simulink model "TosAeb"  (R2021a)
settings: Solver=FixedStepDiscrete  StartTime=0.0  StopTime=inf  FixedStep=0.01  RelTol=1e-3
undefined symbols (not defined in the file or in its folder): ACCModSts, AccSts1, AEB_ObjectMovingOrientation, AEB_ObjectMovingState, CN_BTCActive, CN_CurrentGearR, CN_CurrentGearR_DHT, CN_PTCActive, CN_VDCActive, CN_VSC_EspOff, CN_VSC_FCW_AEB_Error, CN_VSC_FCW_AEB_NoDispay, CN_VSC_FCW_AEB_PerfmDgdtn, CN_VSC_FCW_AEB_TempUnAvlbl, CN_VSC_VehStandstill, CNaccelAvoidMax, CNaccelAvoidMin, CNbrakePedalPress, CNcomfortStrLeftAccelMax, CNcomfortStrLeftAccelMin, CNcomfortStrRightAccelMax, CNcomfortStrRightAccelMin, CNDrvDoorOpen, CNDrvModeEco, CNDrvModeNorm, CNDrvModeOther, CNDrvModeSport, CNDrvSBRreminder, CNNull, CNobjInvalidID, CNPassDoorOpen, CNPassSBRreminder, CNPEBSAccelNegInf, CNPEBSAccelNull, CNPEBSTimeInf, CNPEBSTimeNull, CNSTOPMAX, CNSTOPNULL, CNsubjWidth, CNSwtOFF, CNSwtON, CNTTCMAX, CNTTCNULL, CNVehConfigAEBCycNone, CNVehConfigAEBNone, CNVehConfigAEBPedNone, CNVehConfigFCWNone, CNVehConfigJANone, CollisionSeverity, FALSE, FCWSnvty, JACycObjCalcInfoDefaultParams, JAPedObjCalcInfoDefaultParams, JAVehObjCalcInfoDefaultParams, latCycObjCalcInfoDefaultParams, latPedObjCalcInfoDefaultParams, longObjCalcInfoDefaultParams, ModSts, movingStatus, ObjectType ... (+1112)
libraries: simulink x3031, AEBSFunctionLib x50, Ta_Library x33, AEBSStateMachineLib x23, Mylibrary x12, simulink_extras x7, ObjSelect_lib x3, dspstat3 x2
(part 37 of 102)

== system TosAeb/AEB_Objsel/TA_Processor/PreProcessor/VehiclePreProcessor/PathEstimn_Calculate/For Iterator PahEstimn/ComputeVehicleStateAtT1/c ==
  P1Longitudinal : Inport
  P2Longitudinal : Inport  Port=2
  P3Longitudinal : Inport  Port=3
  P1Lateral : Inport  Port=4
  P2Lateral : Inport  Port=5
  P3Lateral : Inport  Port=6
  Add : Sum  Inputs=-+
  Add1 : Sum  Inputs=+-+
  Add2 : Sum  Inputs=-+
  Add3 : Sum  Inputs=+-+
  Gain{,1,5..6} (4 alike) : Gain  Gain=3
  Gain2 : Gain  Gain=6
  Gain3 : Gain  Gain=12
  Gain4 : Gain  Gain=6
  Gain7 : Gain  Gain=6
  Gain8 : Gain  Gain=12
  Gain9 : Gain
  Terminator : Terminator
  Terminator1 : Terminator
  PosnLgt : Outport
  PosnLat : Outport  Port=2
  VLgt : Outport  Port=3
  VLat : Outport  Port=4
  wires:
  P3Longitudinal/1 -> [P3Longitudinal], PosnLgt/1  "PosnLgt"
  P2Longitudinal/1 -> [P2Longitudinal], Gain/1
  Gain/1 -> Add/1
  Gain1/1 -> Add/2
  [P3Longitudinal] -> Gain1/1
  Add/1 -> VLgt/1  "VLgt"
  P1Longitudinal/1 -> Gain2/1
  Gain2/1 -> Add1/1
  [P2Longitudinal] -> Gain3/1
  Gain3/1 -> Add1/2
  Gain4/1 -> Add1/3
  [P3Longitudinal] -> Gain4/1
  Add1/1 -> Terminator/1  "ALgt"
  P3Lateral/1 -> [P3Lateral], PosnLat/1
  P2Lateral/1 -> [P2Lateral], Gain5/1
  Gain5/1 -> Add2/1
  Add2/1 -> VLat/1  "VLat"
  [P3Lateral] -> Gain6/1
  Gain6/1 -> Add2/2
  P1Lateral/1 -> Gain7/1
  Gain7/1 -> Add3/1
  [P2Lateral] -> Gain8/1
  Gain8/1 -> Add3/2
  Gain9/1 -> Add3/3
  [P3Lateral] -> Gain9/1
  Add3/1 -> Terminator1/1  "ALgt"

== system TosAeb/AEB_Objsel/TA_Processor/PreProcessor/VehiclePreProcessor/PathEstimn_Calculate/For Iterator PahEstimn/InitialControlPointsCalculation/InitialControlPointsCalculation ==
  PositionLongitudinal : Inport
  VelocityLongitudinal : Inport  Port=2
  AccelerationLongitudinal : Inport  Port=3
  PositionLateral : Inport  Port=4
  VelocityLateral : Inport  Port=5
  AccelerationLateral : Inport  Port=6
  StoppingDistances : Inport  Port=7
  Add{,1..2,4} (4 alike) : Sum
  Add3 : Sum  Inputs=+-+
  Add5 : Sum  Inputs=+-+
  Bus Creator : BusCreator  Inputs=6
  Bus Selector : BusSelector  OutputSignals=StopsWithinSegment,LongitudinalStoppingDistance,LateralStoppingDistance
  Gain : Gain  Gain=single(1/3)
  Gain1 : Gain  Gain=2
  Gain2 : Gain  Gain=single(1/3)
  Gain3 : Gain  Gain=single(1/6)
  Gain4 : Gain  Gain=single(1/6)
  Gain5 : Gain  Gain=2
  Signal Conversion : SignalConversion  OverrideOpt=off
  Signal Conversion1 : SignalConversion  OverrideOpt=off
  Switch{,1..3} (4 alike) : Switch  Criteria=u2 ~= 0
  InitialControlPoints : Outport
  wires:
  PositionLongitudinal/1 -> [PosnLgt], Add/1, Signal Conversion/1
  VelocityLongitudinal/1 -> Gain/1
  Gain/1 -> Add/2
  Add/1 -> Gain1/1, Switch/3
  StoppingDistances/1 -> Bus Selector/1
  Bus Selector/1 -> [StopsWithinSegment]  "<StopsWithinSegment>"
  Bus Selector/2 -> Add1/1  "<LongitudinalStoppingDistance>"
  [PosnLgt] -> Add1/2
  Add1/1 -> [StopPosnLgt]
  Bus Selector/3 -> Add2/1  "<LateralStoppingDistance>"
  PositionLateral/1 -> Add4/1, Signal Conversion1/1, [PosnLat]
  [PosnLat] -> Add2/2
  Add2/1 -> [StopPosnLat]
  [StopPosnLgt] -> Switch/1
  [StopsWithinSegment] -> Switch/2
  Gain1/1 -> Add3/1
  [PosnLgt] -> Add3/2
  Add3/1 -> Switch1/3
  [StopPosnLgt] -> Switch1/1
  [StopsWithinSegment] -> Switch1/2
  AccelerationLongitudinal/1 -> Gain3/1
  Gain3/1 -> Add3/3
  Gain2/1 -> Add4/2
  VelocityLateral/1 -> Gain2/1
  AccelerationLateral/1 -> Gain4/1
  Gain4/1 -> Add5/3
  [PosnLat] -> Add5/2
  Add4/1 -> Switch3/3, Gain5/1
  Add5/1 -> Switch2/3
  [StopPosnLat] -> Switch2/1
  [StopsWithinSegment] -> Switch2/2
  Gain5/1 -> Add5/1
  [StopPosnLat] -> Switch3/1
  [StopsWithinSegment] -> Switch3/2
  Signal Conversion/1 -> Bus Creator/1  "P0Longitudinal"
  Switch2/1 -> Bus Creator/6  "P2Lateral"
  Switch/1 -> Bus Creator/2  "P1Longitudinal"
  Switch1/1 -> Bus Creator/3  "P2Longitudinal"
  Signal Conversion1/1 -> Bus Creator/4  "P0Lateral"
  Switch3/1 -> Bus Creator/5  "P1Lateral"
  Bus Creator/1 -> InitialControlPoints/1  "InitialControlPoints"

== system TosAeb/FcwAebJa_Step_sys/ALG_AEBSystem/ALG_AEBController/AEB_FuncCondCalcApp/CycFunctionCondCalc/cycAEBfullSuppAborCond/cycAEBfullAborCondNoStandstillCalc/cycAEBfullAborCondFinalJudge ==
  cycAEBfullObjRelAborCondFulfilled : Inport
  cycAEBfullVehRelAborCondFulfilled : Inport  Port=2
  cycAEBfullSituationRelAborCondFulfilled : Inport  Port=3
  Compare To Constant1 : simulink/Logic and Bit Operations/Compare To Constant  relop===  const=TRUE  OutDataTypeStr=boolean
  Compare To Constant2 : simulink/Logic and Bit Operations/Compare To Constant  relop===  const=TRUE  OutDataTypeStr=boolean
  Compare To Constant3 : simulink/Logic and Bit Operations/Compare To Constant  relop===  const=TRUE  OutDataTypeStr=boolean
  Logical Operator : Logic  Operator=OR  Inputs=3  AllPortsSameDT=off  OutDataTypeStr=boolean
  cycAEBfullAborCondNoStandstillFulfilled : Outport
  wires:
  Compare To Constant3/1 -> Logical Operator/1
  cycAEBfullVehRelAborCondFulfilled/1 -> Compare To Constant1/1
  cycAEBfullSituationRelAborCondFulfilled/1 -> Compare To Constant2/1
  Logical Operator/1 -> cycAEBfullAborCondNoStandstillFulfilled/1  "cycAEBfullAborCondNoStandstillFulfilled"
  cycAEBfullObjRelAborCondFulfilled/1 -> Compare To Constant3/1
  Compare To Constant1/1 -> Logical Operator/2
  Compare To Constant2/1 -> Logical Operator/3

== system TosAeb/FcwAebJa_Step_sys/ALG_AEBSystem/ALG_AEBController/AEB_FuncCondCalcApp/CycFunctionCondCalc/cycAEBfullSuppAborCond/cycAEBfullAborCondNoStandstillCalc/cycAEBfullObjRelActiveCondCalc ==
  ObjectID : Inport
  objIsFusionTarget : Inport  Port=2
  latCollsionRiskProb : Inport  Port=3
  Chart : Stateflow chart  TreatAsAtomicUnit=on
  Compare To Constant : simulink/Logic and Bit Operations/Compare To Constant  relop===  const=TRUE  OutDataTypeStr=boolean
  Constant : Constant  Value=P_AEB_Func_cycAEBfulColliisonRiskProbAborThresObjID
  Unit Delay : UnitDelay  SampleTime=-1  HasFrameUpgradeWarning=on
  cycAEBfullObjRelAborCondFulfilled : Outport
  wires:
  Constant/1 -> Chart/5(P_cycAEBfulColliisonRiskProbAborThresObjID)
  latCollsionRiskProb/1 -> Chart/4(objLatCollisionProb)
  Unit Delay/1 -> Chart/2(targetObjIDObjCalcK1)
  Chart/1(cycAEBfullObjRelAborCondFulfilled) -> cycAEBfullObjRelAborCondFulfilled/1
  ObjectID/1 -> Unit Delay/1, Chart/1(targetObjID)
  objIsFusionTarget/1 -> Compare To Constant/1
  Compare To Constant/1 -> Chart/3(objIsFusionTarget)

== system TosAeb/FcwAebJa_Step_sys/ALG_AEBSystem/ALG_AEBController/AEB_FuncCondCalcApp/CycFunctionCondCalc/cycAEBfullSuppAborCond/cycAEBfullAborCondNoStandstillCalc/cycAEBfullSituationRelAborCondCalc ==
  cycVehVelReduced : Inport
  cycCollisionSeverity : Inport  Port=2
  objLatCollsionRiskProb : Inport  Port=3
  subjVel : Inport  Port=4
  dy : Inport  Port=5
  dx : Inport  Port=6
  ObjectMovingState : Inport  Port=7
  ObjLatMovContiInvalidCalc : Subsystem
  Subsystem : Subsystem
  cycAEBfullSituationRelAborCondFulfilled : Outport
  wires:
  ObjectMovingState/1 -> ObjLatMovContiInvalidCalc/4(ObjectMovingState)
  dx/1 -> ObjLatMovContiInvalidCalc/3(dx)
  dy/1 -> ObjLatMovContiInvalidCalc/2(dy)
  subjVel/1 -> ObjLatMovContiInvalidCalc/1(subjVel)
  Subsystem/1(cycAEBfullSituationRelAborCondFulfilled) -> cycAEBfullSituationRelAborCondFulfilled/1
  ObjLatMovContiInvalidCalc/1(ObjLatMovContiInvalid) -> Subsystem/4(ObjLatMovContiInvalid)
  objLatCollsionRiskProb/1 -> Subsystem/3(objLatCollsionRiskProb)
  cycVehVelReduced/1 -> Subsystem/2(cycVehVelReduced)
  cycCollisionSeverity/1 -> Subsystem/1(cycCollisionSeverity)

== system TosAeb/FcwAebJa_Step_sys/ALG_AEBSystem/ALG_AEBController/AEB_FuncCondCalcApp/CycFunctionCondCalc/cycAEBfullSuppAborCond/cycAEBfullAborCondNoStandstillCalc/cycAEBfullVehRelAborCondCalc ==
  note: 取消自车速度判断条件
  SteerWheelAngle : Inport
  SteerWheelSpd : Inport  Port=2
  vehStateCalcAEBfullAborFlg : Inport  Port=3
  cycAEBfullWorkTime : Inport  Port=4
  vehStateCalcStandstill : Inport  Port=5
  Abs : Abs
  Abs1 : Abs
  Compare To Constant : simulink/Logic and Bit Operations/Compare To Constant  relop=>  const=P_AEB_Func_cycAEBfullStrWheelAngleAborThres  OutDataTypeStr=boolean
  Compare To Constant1 : simulink/Logic and Bit Operations/Compare To Constant  relop=>  const=P_AEB_Func_cycAEBfullStrWheelAngleSpdAborThres  OutDataTypeStr=boolean
  Compare To Constant2 : simulink/Logic and Bit Operations/Compare To Constant  relop=>  const=P_AEB_Func_cycAEBfullActivationTimeAborThres  OutDataTypeStr=boolean
  Compare To Constant3 : simulink/Logic and Bit Operations/Compare To Constant  relop===  const=TRUE  OutDataTypeStr=boolean
  Compare To Constant4 : simulink/Logic and Bit Operations/Compare To Constant  relop===  const=FALSE  OutDataTypeStr=boolean
  Logical Operator : Logic  Operator=OR  AllPortsSameDT=off  OutDataTypeStr=boolean
  Logical Operator2 : Logic  Operator=OR  Inputs=3  AllPortsSameDT=off  OutDataTypeStr=boolean
  Logical Operator4 : Logic  AllPortsSameDT=off  OutDataTypeStr=boolean
  cycAEBfullVehRelAborCondFulfilled : Outport
  wires:
  Compare To Constant2/1 -> Logical Operator4/2
  Compare To Constant4/1 -> Logical Operator4/1
  vehStateCalcStandstill/1 -> Compare To Constant4/1
  Logical Operator2/1 -> cycAEBfullVehRelAborCondFulfilled/1  "cycAEBfullVehRelAborCondFulfilled"
  Logical Operator/1 -> Logical Operator2/1  "strReleventAborCondFulfilled"
  Compare To Constant/1 -> Logical Operator/1
  vehStateCalcAEBfullAborFlg/1 -> Compare To Constant3/1
  cycAEBfullWorkTime/1 -> Compare To Constant2/1
  SteerWheelAngle/1 -> Abs/1
  Compare To Constant1/1 -> Logical Operator/2
  Abs1/1 -> Compare To Constant1/1
  SteerWheelSpd/1 -> Abs1/1
  Abs/1 -> Compare To Constant/1
  Logical Operator4/1 -> Logical Operator2/3  "cycAEBfullWorkTimeRelCondFulfilled"
  Compare To Constant3/1 -> Logical Operator2/2  "vehStateCalcAEBfullAborFlg"

== system TosAeb/FcwAebJa_Step_sys/ALG_AEBSystem/ALG_AEBController/AEB_FuncCondCalcApp/CycFunctionCondCalc/cycAEBfullSuppAborCond/cycAEBfullAborCondStandstillCalc/cycAEBfullVehRelAborCondCalc ==
  note: 取消速度限制
  subjVel : Inport
  SteerWheelAngle : Inport  Port=2
  SteerWheelSpd : Inport  Port=3
  vehStateCalcAEBfullAborFlg : Inport  Port=4
  cycAEBfullWorkTime : Inport  Port=5
  vehStateCalcStandstill : Inport  Port=6
  Abs : Abs
  Abs1 : Abs
  Compare To Constant : simulink/Logic and Bit Operations/Compare To Constant  relop=>  const=1.57  OutDataTypeStr=boolean
  Compare To Constant1 : simulink/Logic and Bit Operations/Compare To Constant  relop=>  const=1.75  OutDataTypeStr=boolean
  Compare To Constant2 : simulink/Logic and Bit Operations/Compare To Constant  relop=>  const=3.5  OutDataTypeStr=boolean
  Compare To Constant3 : simulink/Logic and Bit Operations/Compare To Constant  relop===  const=TRUE  OutDataTypeStr=boolean
  Compare To Constant4 : simulink/Logic and Bit Operations/Compare To Constant  relop===  const=TRUE  OutDataTypeStr=boolean
  Compare To Constant7 : simulink/Logic and Bit Operations/Compare To Constant  relop=<  const=1.5  OutDataTypeStr=boolean
  Logical Operator : Logic  Operator=OR  AllPortsSameDT=off  OutDataTypeStr=boolean
  Logical Operator2 : Logic  Operator=OR  Inputs=3  AllPortsSameDT=off  OutDataTypeStr=boolean
  Logical Operator4 : Logic  Inputs=3  AllPortsSameDT=off  OutDataTypeStr=boolean
  cycAEBfullVehRelAborCondFulfilled : Outport
  wires:
  Compare To Constant2/1 -> Logical Operator4/3
  Compare To Constant7/1 -> Logical Operator4/1
  Compare To Constant4/1 -> Logical Operator4/2
  Compare To Constant3/1 -> Logical Operator2/2  "vehStateCalcAEBfullAborFlg"
  Abs/1 -> Compare To Constant/1
  SteerWheelSpd/1 -> Abs1/1
  Abs1/1 -> Compare To Constant1/1
  Compare To Constant1/1 -> Logical Operator/2
  SteerWheelAngle/1 -> Abs/1
  cycAEBfullWorkTime/1 -> Compare To Constant2/1
  vehStateCalcAEBfullAborFlg/1 -> Compare To Constant3/1
  Compare To Constant/1 -> Logical Operator/1
  Logical Operator/1 -> Logical Operator2/1  "strReleventAborCondFulfilled"
  Logical Operator2/1 -> cycAEBfullVehRelAborCondFulfilled/1  "cycAEBfullVehRelAborCondFulfilled"
  subjVel/1 -> Compare To Constant7/1
  vehStateCalcStandstill/1 -> Compare To Constant4/1
  Logical Operator4/1 -> Logical Operator2/3  "cycAEBfullWorkTimeRelCondFulfilled"

== system TosAeb/FcwAebJa_Step_sys/ALG_AEBSystem/ALG_AEBController/AEB_FuncCondCalcApp/CycFunctionCondCalc/cycAEBfullSuppAborCond/cycAEBfullSuppCondCalc/cycAEBfullObjRelSuppCondCalc ==
  ObjectID : Inport
  vxAbsObj : Inport  Port=2
  ObjFusQuality : Inport  Port=3
  dy : Inport  Port=4
  vy : Inport  Port=5
  Abs : Abs
  Compare To Constant : simulink/Logic and Bit Operations/Compare To Constant  relop=>  const=CNNull  OutDataTypeStr=boolean
  Compare To Constant1 : simulink/Logic and Bit Operations/Compare To Constant  relop=>  const=P_AEB_Func_cycAEBfullVxAbsObjSuppThres  OutDataTypeStr=boolean
  Compare To Constant11 : simulink/Logic and Bit Operations/Compare To Constant  relop===  const=FALSE  OutDataTypeStr=boolean
  Compare To Constant2 : simulink/Logic and Bit Operations/Compare To Constant  relop=>  const=CNNull  OutDataTypeStr=boolean
  Compare To Constant3 : simulink/Logic and Bit Operations/Compare To Constant  relop=<  const=CNNull  OutDataTypeStr=boolean
  Compare To Constant4 : simulink/Logic and Bit Operations/Compare To Constant  relop===  const=CNobjInvalidID  OutDataTypeStr=boolean
  Compare To Constant5 : simulink/Logic and Bit Operations/Compare To Constant  relop=<  const=CNNull  OutDataTypeStr=boolean
  Logical Operator1 : Logic  AllPortsSameDT=off  OutDataTypeStr=boolean
  Logical Operator2 : Logic  AllPortsSameDT=off  OutDataTypeStr=boolean
  Logical Operator3 : Logic  Operator=OR  AllPortsSameDT=off  OutDataTypeStr=boolean
  Logical Operator4 : Logic  Operator=OR  Inputs=3  AllPortsSameDT=off  OutDataTypeStr=boolean
  Terminator : Terminator
  cycAEBfullObjRelSuppCondFulfilled : Outport
  wires:
  ObjFusQuality/1 -> Compare To Constant11/1
  vy/1 -> Compare To Constant2/1, Compare To Constant5/1
  Compare To Constant2/1 -> Logical Operator1/2
  dy/1 -> Compare To Constant/1, Compare To Constant3/1
  Logical Operator2/1 -> Logical Operator3/2
  Compare To Constant/1 -> Logical Operator1/1
  Compare To Constant3/1 -> Logical Operator2/1
  Logical Operator1/1 -> Logical Operator3/1
  Compare To Constant5/1 -> Logical Operator2/2
  Compare To Constant11/1 -> Logical Operator4/3  "ObjFusQuality"
  Logical Operator4/1 -> cycAEBfullObjRelSuppCondFulfilled/1
  vxAbsObj/1 -> Abs/1
  Compare To Constant1/1 -> Logical Operator4/2
  Abs/1 -> Compare To Constant1/1
  ObjectID/1 -> Compare To Constant4/1
  Compare To Constant4/1 -> Logical Operator4/1
  Logical Operator3/1 -> Terminator/1

== system TosAeb/FcwAebJa_Step_sys/ALG_AEBSystem/ALG_AEBController/AEB_FuncCondCalcApp/CycFunctionCondCalc/cycAEBfullSuppAborCond/cycAEBfullSuppCondCalc/cycAEBfullSituationRelSuppCondCalc ==
  latCollisionSeverity : Inport
  subjCurvTraj : Inport  Port=2
  subjLatAccel : Inport  Port=3
  cycCollisionRisk : Inport  Port=4
  cycCollisionProbFlg : Inport  Port=5
  ObjectTypeCameraConfirmed : Inport  Port=6
  vy : Inport  Port=7
  dxFrontBumper : Inport  Port=8
  dy : Inport  Port=9
  FcwHMISetting : Inport  Port=10
  subVel : Inport  Port=11
  distBraking4FullBrake : Inport  Port=12
  distSecure : Inport  Port=13
  DistanceBelowMinimumForFullBrakeCalc : Subsystem
  DistanceTriggerForBarkingCalc : Subsystem
  LatDistCond4fullBrakeCalc : Subsystem
  Subsystem : Subsystem
  objLatVyCond4FullBrakeCalc : Subsystem
  cycAEBfullSituationRelSuppCondFulfilled : Outport
  wires:
  DistanceTriggerForBarkingCalc/1(objLongDistCond4FullBrake) -> Subsystem/10(objLongDistCond4FullBrake)
  DistanceBelowMinimumForFullBrakeCalc/1(distMinCond4Brake) -> Subsystem/9(distMinCond4Brake)
  LatDistCond4fullBrakeCalc/1(latDistCond4fullBrake) -> Subsystem/8(latDistCond4fullBrake)
  objLatVyCond4FullBrakeCalc/1(objLatVyCond) -> Subsystem/7(objLatVyCond)
  distSecure/1 -> DistanceTriggerForBarkingCalc/3(distSecure)
  distBraking4FullBrake/1 -> DistanceTriggerForBarkingCalc/2(distBraking4FullBrake)
  Subsystem/1(cycAEBfullSituationRelSuppCondFulfilled) -> cycAEBfullSituationRelSuppCondFulfilled/1
  cycCollisionProbFlg/1 -> Subsystem/5(cycCollisionProbFlg)
  ObjectTypeCameraConfirmed/1 -> objLatVyCond4FullBrakeCalc/1(ObjectTypeCameraConfirmed), Subsystem/6(ObjectTypeCameraConfirmed)
  subjLatAccel/1 -> Subsystem/3(subjLatAccel)
  subjCurvTraj/1 -> Subsystem/2(subjCurvTraj)
  cycCollisionRisk/1 -> Subsystem/4(cycCollisionRisk)
  latCollisionSeverity/1 -> Subsystem/1(latCollisionSeverity)
  subVel/1 -> DistanceBelowMinimumForFullBrakeCalc/2(subVel)
  dy/1 -> LatDistCond4fullBrakeCalc/1(dy)
  FcwHMISetting/1 -> LatDistCond4fullBrakeCalc/2(FcwHMISetting)
  dxFrontBumper/1 -> DistanceBelowMinimumForFullBrakeCalc/1(dxFrontBumper), DistanceTriggerForBarkingCalc/1(dxFrontBumper)
  vy/1 -> objLatVyCond4FullBrakeCalc/2(vyCorr), LatDistCond4fullBrakeCalc/3(vy)

== system TosAeb/FcwAebJa_Step_sys/ALG_AEBSystem/ALG_AEBController/AEB_FuncCondCalcApp/CycFunctionCondCalc/cycAEBfullSuppAborCond/cycAEBfullSuppCondCalc/cycAEBfullSuppCondFinalJudge ==
  cycAEBfullObjRelSuppCondFulfilled : Inport
  cycAEBfullVehRelSuppCondFulfilled : Inport  Port=2
  cycAEBfullSituationRelSuppCondFulfilled : Inport  Port=3
  Compare To Constant : simulink/Logic and Bit Operations/Compare To Constant  relop===  const=TRUE  OutDataTypeStr=boolean
  Compare To Constant1 : simulink/Logic and Bit Operations/Compare To Constant  relop===  const=TRUE  OutDataTypeStr=boolean
  Compare To Constant2 : simulink/Logic and Bit Operations/Compare To Constant  relop===  const=TRUE  OutDataTypeStr=boolean
  Logical Operator : Logic  Operator=OR  Inputs=3  AllPortsSameDT=off  OutDataTypeStr=boolean
  cycAEBfullSuppCondFulfilled : Outport
  wires:
  Compare To Constant2/1 -> Logical Operator/3
  Compare To Constant1/1 -> Logical Operator/2
  Compare To Constant/1 -> Logical Operator/1
  cycAEBfullVehRelSuppCondFulfilled/1 -> Compare To Constant1/1
  cycAEBfullObjRelSuppCondFulfilled/1 -> Compare To Constant/1
  cycAEBfullSituationRelSuppCondFulfilled/1 -> Compare To Constant2/1
  Logical Operator/1 -> cycAEBfullSuppCondFulfilled/1

== system TosAeb/FcwAebJa_Step_sys/ALG_AEBSystem/ALG_AEBController/AEB_FuncCondCalcApp/CycFunctionCondCalc/cycAEBfullSuppAborCond/cycAEBfullSuppCondCalc/cycAEBfullVehRelSuppCondCalc ==
  subjVel : Inport
  VehConfigIbooster : Inport  Port=2
  ESP_MasterCylindBrakePressValid : Inport  Port=3
  ESP_MasterCylindBrakePress : Inport  Port=4
  SteerWheelAngle : Inport  Port=5
  SteerWheelSpd : Inport  Port=6
  AccrCyclPosnDiagc : Inport  Port=7
  vehStateCalcCycAEBfullSuppFlg : Inport  Port=8
  Abs : Abs
  Abs1 : Abs
  AccReleventSuppCondDelayAbortion : AEBSFunctionLib/StateDelayAbortion  SystemSampleTime=-1  VariantActivationTime=update diagram  Latency=0  AutoFrameSizeCalculation=off
  Compare To Constant : simulink/Logic and Bit Operations/Compare To Constant  relop=>  const=P_AEB_Func_cycAEBfullStrWheelAngleSupThres  OutDataTypeStr=boolean
  Compare To Constant1 : simulink/Logic and Bit Operations/Compare To Constant  relop=>  const=P_AEB_Func_cycAEBfullGasPedalSupThres  OutDataTypeStr=boolean
  Compare To Constant2 : simulink/Logic and Bit Operations/Compare To Constant  relop=>  const=P_AEB_Func_cycAEBfullStrWheelAngleSpdSupThres  OutDataTypeStr=boolean
  Compare To Constant7 : simulink/Logic and Bit Operations/Compare To Constant  relop===  const=TRUE  OutDataTypeStr=boolean
  Compare To Constant8 : simulink/Logic and Bit Operations/Compare To Constant  relop=>  const=P_AEB_Func_cycAEBfullSubjVelMax  OutDataTypeStr=boolean
  Compare To Constant9 : simulink/Logic and Bit Operations/Compare To Constant  relop=<  const=P_AEB_Func_cycAEBfullSubjVelMin  OutDataTypeStr=boolean
  Constant : Constant  Value=P_AEB_Func_cycAEBfullStrCondAborDelayTime
  Constant1 : Constant  Value=0.02
  Constant2 : Constant  Value=P_AEB_Func_cycAEBfullAccrCondAborDelayTime
  Constant3 : Constant  Value=0.02
  Logical Operator : Logic  Operator=OR  AllPortsSameDT=off  OutDataTypeStr=boolean
  Logical Operator4 : Logic  Operator=OR  AllPortsSameDT=off  OutDataTypeStr=boolean
  Logical Operator5 : Logic  Operator=OR  Inputs=5  AllPortsSameDT=off  OutDataTypeStr=boolean
  StrReleventSuppCondDelayAbortion : AEBSFunctionLib/StateDelayAbortion  SystemSampleTime=-1  VariantActivationTime=update diagram  Latency=0  AutoFrameSizeCalculation=off
  brakeReleventSuppCond : Subsystem
  cycAEBfullVehRelSuppCondFulfilled : Outport
  wires:
  VehConfigIbooster/1 -> brakeReleventSuppCond/1(VehConfigIbooster)
  ESP_MasterCylindBrakePress/1 -> brakeReleventSuppCond/3(ESP_MasterCylindBrakePress)
  ESP_MasterCylindBrakePressValid/1 -> brakeReleventSuppCond/2(ESP_MasterCylindBrakePressValid)
  Compare To Constant1/1 -> AccReleventSuppCondDelayAbortion/1
  Constant2/1 -> AccReleventSuppCondDelayAbortion/2
  Constant3/1 -> AccReleventSuppCondDelayAbortion/3
  AccrCyclPosnDiagc/1 -> Compare To Constant1/1
  Logical Operator4/1 -> StrReleventSuppCondDelayAbortion/1  "strReleventSuppCondFulfilled"
  Constant1/1 -> StrReleventSuppCondDelayAbortion/3
  Constant/1 -> StrReleventSuppCondDelayAbortion/2
  AccReleventSuppCondDelayAbortion/1 -> Logical Operator5/4  "accReleventSuppCondFulfilled"
  Abs1/1 -> Compare To Constant2/1
  vehStateCalcCycAEBfullSuppFlg/1 -> Compare To Constant7/1
  Compare To Constant8/1 -> Logical Operator/1
  Compare To Constant2/1 -> Logical Operator4/2
  SteerWheelSpd/1 -> Abs1/1
  Compare To Constant/1 -> Logical Operator4/1
  Abs/1 -> Compare To Constant/1
  SteerWheelAngle/1 -> Abs/1
  StrReleventSuppCondDelayAbortion/1 -> Logical Operator5/3  "strReleventSuppCondFulfilled"
  brakeReleventSuppCond/1(brakeReleventSuppCond) -> Logical Operator5/2  "BrkReleventSuppCondFulfilled"
  Compare To Constant9/1 -> Logical Operator/2
  Compare To Constant7/1 -> Logical Operator5/5  "vehStateCalcPedPrefillSuppCondFulfilled"
  Logical Operator/1 -> Logical Operator5/1  "subjVelReleventSuppCondFulfilled"
  subjVel/1 -> Compare To Constant9/1, Compare To Constant8/1
  Logical Operator5/1 -> cycAEBfullVehRelSuppCondFulfilled/1  "pedAEBfullVehRelSuppCondFulfilled"

== system TosAeb/FcwAebJa_Step_sys/ALG_AEBSystem/ALG_AEBController/AEB_FuncCondCalcApp/CycFunctionCondCalc/cycAEBpartSuppAborCond/cycAEBpartAborCondNoStandstillCalc/cycAEBpartAborCondFinalJudge ==
  cycAEBpartObjRelAborCondFulfilled : Inport
  cycAEBpartVehRelAborCondFulfilled : Inport  Port=2
  cycAEBpartSituationRelAborCondFulfilled : Inport  Port=3
  Compare To Constant1 : simulink/Logic and Bit Operations/Compare To Constant  relop===  const=TRUE  OutDataTypeStr=boolean
  Compare To Constant2 : simulink/Logic and Bit Operations/Compare To Constant  relop===  const=TRUE  OutDataTypeStr=boolean
  Compare To Constant3 : simulink/Logic and Bit Operations/Compare To Constant  relop===  const=TRUE  OutDataTypeStr=boolean
  Logical Operator : Logic  Operator=OR  Inputs=3  AllPortsSameDT=off  OutDataTypeStr=boolean
  cycAEBpartAborCondNoStandstillFulfilled : Outport
  wires:
  Compare To Constant3/1 -> Logical Operator/1
  cycAEBpartVehRelAborCondFulfilled/1 -> Compare To Constant1/1
  cycAEBpartSituationRelAborCondFulfilled/1 -> Compare To Constant2/1
  Logical Operator/1 -> cycAEBpartAborCondNoStandstillFulfilled/1  "cycAEBpartAborCondNoStandstillFulfilled"
  cycAEBpartObjRelAborCondFulfilled/1 -> Compare To Constant3/1
  Compare To Constant1/1 -> Logical Operator/2
  Compare To Constant2/1 -> Logical Operator/3

== system TosAeb/FcwAebJa_Step_sys/ALG_AEBSystem/ALG_AEBController/AEB_FuncCondCalcApp/CycFunctionCondCalc/cycAEBpartSuppAborCond/cycAEBpartAborCondNoStandstillCalc/cycAEBpartObjRelActiveCondCalc ==
  ObjectID : Inport
  objIsFusionTarget : Inport  Port=2
  latCollsionRiskProb : Inport  Port=3
  Compare To Constant : simulink/Logic and Bit Operations/Compare To Constant  relop===  const=TRUE  OutDataTypeStr=boolean
  Constant : Constant  Value=P_AEB_Func_cycAEBpartColliisonRiskProbAborThresObjID
  Unit Delay : UnitDelay  SampleTime=-1  HasFrameUpgradeWarning=on
  cycAEBpartObjRelAborCondFlow : Stateflow chart  TreatAsAtomicUnit=on
  cycAEBpartObjRelAborCondFulfilled : Outport
  wires:
  Constant/1 -> cycAEBpartObjRelAborCondFlow/5(P_cycAEBpartColliisonRiskProbAborThresObjID)
  latCollsionRiskProb/1 -> cycAEBpartObjRelAborCondFlow/4(objLatCollisionProb)
  Unit Delay/1 -> cycAEBpartObjRelAborCondFlow/2(targetObjIDObjCalcK1)
  cycAEBpartObjRelAborCondFlow/1(cycAEBfullObjRelAborCondFulfilled) -> cycAEBpartObjRelAborCondFulfilled/1
  ObjectID/1 -> Unit Delay/1, cycAEBpartObjRelAborCondFlow/1(targetObjID)
  objIsFusionTarget/1 -> Compare To Constant/1
  Compare To Constant/1 -> cycAEBpartObjRelAborCondFlow/3(objIsFusionTarget)

== system TosAeb/FcwAebJa_Step_sys/ALG_AEBSystem/ALG_AEBController/AEB_FuncCondCalcApp/CycFunctionCondCalc/cycAEBpartSuppAborCond/cycAEBpartAborCondNoStandstillCalc/cycAEBpartSituationRelAborCondCalc ==
  cycVehVelReduced : Inport
  latCollisionSeverity : Inport  Port=2
  CollsionRiskProb : Inport  Port=3
  subjVel : Inport  Port=4
  dy : Inport  Port=5
  dx : Inport  Port=6
  ObjectMovingState : Inport  Port=7
  ObjLatMovContiInvalidCalc : Subsystem
  Subsystem : Subsystem
  cycAEBpartSituationRelAborCondFulfilled : Outport
  wires:
  ObjectMovingState/1 -> ObjLatMovContiInvalidCalc/4(ObjectMovingState)
  dx/1 -> ObjLatMovContiInvalidCalc/3(dx)
  dy/1 -> ObjLatMovContiInvalidCalc/2(dy)
  subjVel/1 -> ObjLatMovContiInvalidCalc/1(subjVel)
  ObjLatMovContiInvalidCalc/1(ObjLatMovContiInvalid) -> Subsystem/4(ObjLatMovContiInvalid)
  Subsystem/1(cycAEBpartSituationRelAborCondFulfilled) -> cycAEBpartSituationRelAborCondFulfilled/1
  CollsionRiskProb/1 -> Subsystem/3(CollsionRiskProb)
  cycVehVelReduced/1 -> Subsystem/2(cycVehVelReduced)
  latCollisionSeverity/1 -> Subsystem/1(latCollisionSeverity)

== system TosAeb/FcwAebJa_Step_sys/ALG_AEBSystem/ALG_AEBController/AEB_FuncCondCalcApp/CycFunctionCondCalc/cycAEBpartSuppAborCond/cycAEBpartAborCondNoStandstillCalc/cycAEBpartVehRelAborCondCalc ==
  note: 取消自车速度判断条件
  SteerWheelAngle : Inport
  SteerWheelSpd : Inport  Port=2
  vehStateCalcAEBpartAborFlg : Inport  Port=3
  Abs : Abs
  Abs1 : Abs
  Compare To Constant : simulink/Logic and Bit Operations/Compare To Constant  relop=>  const=P_AEB_Func_cycAEBpartStrWheelAngleAborThres  OutDataTypeStr=boolean
  Compare To Constant1 : simulink/Logic and Bit Operations/Compare To Constant  relop=>  const=P_AEB_Func_cycAEBpartStrWheelAngleSpdAborThres  OutDataTypeStr=boolean
  Compare To Constant3 : simulink/Logic and Bit Operations/Compare To Constant  relop===  const=TRUE  OutDataTypeStr=boolean
  Logical Operator : Logic  Operator=OR  AllPortsSameDT=off  OutDataTypeStr=boolean
  Logical Operator2 : Logic  Operator=OR  AllPortsSameDT=off  OutDataTypeStr=boolean
  cycAEBpartVehRelAborCondFulfilled : Outport
  wires:
  Logical Operator2/1 -> cycAEBpartVehRelAborCondFulfilled/1  "cycAEBpartVehRelAborCondFulfilled"
  Logical Operator/1 -> Logical Operator2/1  "strReleventAborCondFulfilled"
  Compare To Constant/1 -> Logical Operator/1
  vehStateCalcAEBpartAborFlg/1 -> Compare To Constant3/1
  SteerWheelAngle/1 -> Abs/1
  Compare To Constant1/1 -> Logical Operator/2
  Abs1/1 -> Compare To Constant1/1
  SteerWheelSpd/1 -> Abs1/1
  Abs/1 -> Compare To Constant/1
  Compare To Constant3/1 -> Logical Operator2/2  "vehStateCalcAEBfullAborFlg"

== system TosAeb/FcwAebJa_Step_sys/ALG_AEBSystem/ALG_AEBController/AEB_FuncCondCalcApp/CycFunctionCondCalc/cycAEBpartSuppAborCond/cycAEBpartAborCondStandstillCalc/cycAEBpartVehRelAborCondCalc ==
  note: 取消速度限制
  SteerWheelAngle : Inport
  SteerWheelSpd : Inport  Port=2
  vehStateCalcAEBpartAborFlg : Inport  Port=3
  Abs : Abs
  Abs1 : Abs
  Compare To Constant : simulink/Logic and Bit Operations/Compare To Constant  relop=>  const=P_AEB_Func_cycAEBpartStrWheelAngleAborThresStandstill  OutDataTypeStr=boolean
  Compare To Constant1 : simulink/Logic and Bit Operations/Compare To Constant  relop=>  const=P_AEB_Func_cycAEBpartStrWheelAngleSpdAborThresStandstill  OutDataTypeStr=boolean
  Compare To Constant3 : simulink/Logic and Bit Operations/Compare To Constant  relop===  const=TRUE  OutDataTypeStr=boolean
  Logical Operator : Logic  Operator=OR  AllPortsSameDT=off  OutDataTypeStr=boolean
  Logical Operator2 : Logic  Operator=OR  AllPortsSameDT=off  OutDataTypeStr=boolean
  cycAEBpartVehRelAborCondFulfilled : Outport
  wires:
  Compare To Constant3/1 -> Logical Operator2/2  "vehStateCalcAEBfullAborFlg"
  Abs/1 -> Compare To Constant/1
  SteerWheelSpd/1 -> Abs1/1
  Abs1/1 -> Compare To Constant1/1
  Compare To Constant1/1 -> Logical Operator/2
  SteerWheelAngle/1 -> Abs/1
  vehStateCalcAEBpartAborFlg/1 -> Compare To Constant3/1
  Compare To Constant/1 -> Logical Operator/1
  Logical Operator/1 -> Logical Operator2/1  "strReleventAborCondFulfilled"
  Logical Operator2/1 -> cycAEBpartVehRelAborCondFulfilled/1  "cycAEBpartVehRelAborCondFulfilled"

== system TosAeb/FcwAebJa_Step_sys/ALG_AEBSystem/ALG_AEBController/AEB_FuncCondCalcApp/CycFunctionCondCalc/cycAEBpartSuppAborCond/cycAEBpartSuppCondCalc/cycAEBpartObjRelActiveCondCalc ==
  ObjectID : Inport
  vxAbsObj : Inport  Port=2
  ObjFusQuality : Inport  Port=3
  dy : Inport  Port=4
  vy : Inport  Port=5
  Abs : Abs
  Compare To Constant : simulink/Logic and Bit Operations/Compare To Constant  relop=>  const=CNNull  OutDataTypeStr=boolean
  Compare To Constant1 : simulink/Logic and Bit Operations/Compare To Constant  relop=>  const=P_AEB_Func_cycAEBpartVxAbsObjSuppThres  OutDataTypeStr=boolean
  Compare To Constant11 : simulink/Logic and Bit Operations/Compare To Constant  relop===  const=FALSE  OutDataTypeStr=boolean
  Compare To Constant2 : simulink/Logic and Bit Operations/Compare To Constant  relop=>  const=CNNull  OutDataTypeStr=boolean
  Compare To Constant3 : simulink/Logic and Bit Operations/Compare To Constant  relop=<  const=CNNull  OutDataTypeStr=boolean
  Compare To Constant4 : simulink/Logic and Bit Operations/Compare To Constant  relop===  const=CNobjInvalidID  OutDataTypeStr=boolean
  Compare To Constant5 : simulink/Logic and Bit Operations/Compare To Constant  relop=<  const=CNNull  OutDataTypeStr=boolean
  Logical Operator : Logic  Operator=OR  Inputs=3  AllPortsSameDT=off  OutDataTypeStr=boolean
  Logical Operator1 : Logic  AllPortsSameDT=off  OutDataTypeStr=boolean
  Logical Operator2 : Logic  AllPortsSameDT=off  OutDataTypeStr=boolean
  Logical Operator3 : Logic  Operator=OR  AllPortsSameDT=off  OutDataTypeStr=boolean
  Terminator : Terminator
  cycAEBpartObjRelSuppCondFulfilled : Outport
  wires:
  ObjFusQuality/1 -> Compare To Constant11/1
  vy/1 -> Compare To Constant2/1, Compare To Constant5/1
  Compare To Constant2/1 -> Logical Operator1/2
  dy/1 -> Compare To Constant/1, Compare To Constant3/1
  Logical Operator2/1 -> Logical Operator3/2
  Compare To Constant/1 -> Logical Operator1/1
  Compare To Constant3/1 -> Logical Operator2/1
  Logical Operator1/1 -> Logical Operator3/1
  Compare To Constant5/1 -> Logical Operator2/2
  Compare To Constant11/1 -> Logical Operator/3  "ObjFusQuality"
  Logical Operator/1 -> cycAEBpartObjRelSuppCondFulfilled/1
  Compare To Constant1/1 -> Logical Operator/2
  Compare To Constant4/1 -> Logical Operator/1
  Abs/1 -> Compare To Constant1/1
  vxAbsObj/1 -> Abs/1
  ObjectID/1 -> Compare To Constant4/1
  Logical Operator3/1 -> Terminator/1

== system TosAeb/FcwAebJa_Step_sys/ALG_AEBSystem/ALG_AEBController/AEB_FuncCondCalcApp/CycFunctionCondCalc/cycAEBpartSuppAborCond/cycAEBpartSuppCondCalc/cycAEBpartSituationRelActiveCondCalc ==
  latCollisionSeverity : Inport
  subjCurvTraj : Inport  Port=2
  subjLatAccel : Inport  Port=3
  ObjectTypeCameraConfirmed : Inport  Port=4
  colliisonRiskProb : Inport  Port=5
  PreCollisionFlg : Inport  Port=6
  vy : Inport  Port=7
  dxFrontBumper : Inport  Port=8
  distBraking4PartBrake : Inport  Port=9
  distSecure : Inport  Port=10
  subVel : Inport  Port=11
  dy : Inport  Port=12
  FcwHMISetting : Inport  Port=13
  DistanceBelowMinimumForFullBrakeCalc : Subsystem
  LatDistCond4PartBrakeCalc : Subsystem
  cycAEBpartSituationRelSuppCondCalc : Subsystem
  objLatVyCond4PrefillCalc : Subsystem
  objLongDistCond4PartBrakeCalc : Subsystem
  cycAEBpartSituationRelSuppCondFulfilled : Outport
  wires:
  [vy] -> LatDistCond4PartBrakeCalc/3(vyCorr)
  FcwHMISetting/1 -> LatDistCond4PartBrakeCalc/2(FcwHMISetting)
  dy/1 -> LatDistCond4PartBrakeCalc/1(dy)
  subVel/1 -> DistanceBelowMinimumForFullBrakeCalc/2(subVel)
  distSecure/1 -> objLongDistCond4PartBrakeCalc/3(distSecure)
  distBraking4PartBrake/1 -> objLongDistCond4PartBrakeCalc/2(distBraking4PartBrake)
  dxFrontBumper/1 -> objLongDistCond4PartBrakeCalc/1(dxFrontBumper), DistanceBelowMinimumForFullBrakeCalc/1(dxFrontBumper)
  vy/1 -> objLatVyCond4PrefillCalc/2(vy), [vy]
  objLatVyCond4PrefillCalc/1(objLatVyCond) -> cycAEBpartSituationRelSuppCondCalc/7(objLatVyCond)
  LatDistCond4PartBrakeCalc/1(latDistCond4PartBrake) -> cycAEBpartSituationRelSuppCondCalc/10(latDistCond4PartBrake)
  DistanceBelowMinimumForFullBrakeCalc/1(distMinCond4Brake) -> cycAEBpartSituationRelSuppCondCalc/9(distMinCond4Brake)
  cycAEBpartSituationRelSuppCondCalc/1(cycAEBpartSituationRelSuppCondFulfilled) -> cycAEBpartSituationRelSuppCondFulfilled/1
  ObjectTypeCameraConfirmed/1 -> cycAEBpartSituationRelSuppCondCalc/6(ObjectTypeCameraConfirmed), objLatVyCond4PrefillCalc/1(ObjectTypeCameraConfirmed)
  colliisonRiskProb/1 -> cycAEBpartSituationRelSuppCondCalc/5(colliisonRiskProb)
  objLongDistCond4PartBrakeCalc/1(objLongDistCond4PartBrake) -> cycAEBpartSituationRelSuppCondCalc/8(objLongDistCond4PartBrake)
  PreCollisionFlg/1 -> cycAEBpartSituationRelSuppCondCalc/4(PreCollisionFlg)
  subjLatAccel/1 -> cycAEBpartSituationRelSuppCondCalc/3(subjLatAccel)
  subjCurvTraj/1 -> cycAEBpartSituationRelSuppCondCalc/2(subjCurvTraj)
  latCollisionSeverity/1 -> cycAEBpartSituationRelSuppCondCalc/1(latCollisionSeverity)

== system TosAeb/FcwAebJa_Step_sys/ALG_AEBSystem/ALG_AEBController/AEB_FuncCondCalcApp/CycFunctionCondCalc/cycAEBpartSuppAborCond/cycAEBpartSuppCondCalc/cycAEBpartSuppCondFinalJudge ==
  cycAEBpartObjRelSuppCondFulfilled : Inport
  cycAEBpartVehRelSuppCondFulfilled : Inport  Port=2
  cycAEBpartSituationRelSuppCondFulfilled : Inport  Port=3
  Compare To Constant : simulink/Logic and Bit Operations/Compare To Constant  relop===  const=TRUE  OutDataTypeStr=boolean
  Compare To Constant1 : simulink/Logic and Bit Operations/Compare To Constant  relop===  const=TRUE  OutDataTypeStr=boolean
  Compare To Constant2 : simulink/Logic and Bit Operations/Compare To Constant  relop===  const=TRUE  OutDataTypeStr=boolean
  Logical Operator : Logic  Operator=OR  Inputs=3  AllPortsSameDT=off  OutDataTypeStr=boolean
  cycAEBpartSuppCondFulfilled : Outport
  wires:
  Logical Operator/1 -> cycAEBpartSuppCondFulfilled/1
  cycAEBpartSituationRelSuppCondFulfilled/1 -> Compare To Constant2/1
  cycAEBpartObjRelSuppCondFulfilled/1 -> Compare To Constant/1
  cycAEBpartVehRelSuppCondFulfilled/1 -> Compare To Constant1/1
  Compare To Constant/1 -> Logical Operator/1
  Compare To Constant1/1 -> Logical Operator/2
  Compare To Constant2/1 -> Logical Operator/3

== system TosAeb/FcwAebJa_Step_sys/ALG_AEBSystem/ALG_AEBController/AEB_FuncCondCalcApp/CycFunctionCondCalc/cycAEBpartSuppAborCond/cycAEBpartSuppCondCalc/cycAEBpartVehRelActiveCondCalc ==
  note: 增加OR模块
  subjVel : Inport
  VehConfigIbooster : Inport  Port=2
  ESPmasterCylindBrakePressValid : Inport  Port=3
  ESPmasterCylindBrakePress : Inport  Port=4
  IB_BrkPedalAppld : Inport  Port=5
  SteerWheelAngle : Inport  Port=6
  SteerWheelSpd : Inport  Port=7
  AccrCyclPosnDiagc : Inport  Port=8
  vehStateCalcCycAEBpartSuppFlg : Inport  Port=9
  Abs : Abs
  Abs1 : Abs
  AccReleventSuppCondDelayAbortion : AEBSFunctionLib/StateDelayAbortion  SystemSampleTime=-1  VariantActivationTime=update diagram  Latency=0  AutoFrameSizeCalculation=off
  Compare To Constant : simulink/Logic and Bit Operations/Compare To Constant  relop=>  const=P_AEB_Func_cycAEBpartStrWheelAngleSupThres  OutDataTypeStr=boolean
  Compare To Constant1 : simulink/Logic and Bit Operations/Compare To Constant  relop=>  const=P_AEB_Func_cycAEBpartGasPedalSupThres  OutDataTypeStr=boolean
  Compare To Constant2 : simulink/Logic and Bit Operations/Compare To Constant  relop=>  const=P_AEB_Func_cycAEBpartStrWheelAngleSpdSupThres  OutDataTypeStr=boolean
  Compare To Constant7 : simulink/Logic and Bit Operations/Compare To Constant  relop===  const=TRUE  OutDataTypeStr=boolean
  Compare To Constant8 : simulink/Logic and Bit Operations/Compare To Constant  relop=>  const=P_AEB_Func_cycAEBpartSubjVelMax  OutDataTypeStr=boolean
  Compare To Constant9 : simulink/Logic and Bit Operations/Compare To Constant  relop=<  const=P_AEB_Func_cycAEBpartSubjVelMin  OutDataTypeStr=boolean
  Constant : Constant  Value=P_AEB_Func_cycAEBpartStrCondAborDelayTime
  Constant1 : Constant  Value=0.02
  Constant2 : Constant  Value=P_AEB_Func_cycAEBpartAccrCondAborDelayTime
  Constant3 : Constant  Value=0.02
  Logical Operator : Logic  Operator=OR  AllPortsSameDT=off  OutDataTypeStr=boolean
  Logical Operator4 : Logic  Operator=OR  AllPortsSameDT=off  OutDataTypeStr=boolean
  Logical Operator5 : Logic  Operator=OR  Inputs=5  AllPortsSameDT=off  OutDataTypeStr=boolean
  StrReleventSuppCondDelayAbortion : AEBSFunctionLib/StateDelayAbortion  SystemSampleTime=-1  VariantActivationTime=update diagram  Latency=0  AutoFrameSizeCalculation=off
  brakeReleventSuppCond : Subsystem
  cycAEBpartVehRelSuppCondFulfilled : Outport
  wires:
  VehConfigIbooster/1 -> brakeReleventSuppCond/1(VehConfigIbooster)
  IB_BrkPedalAppld/1 -> brakeReleventSuppCond/4(IB_BrkPedalAppld)
  ESPmasterCylindBrakePress/1 -> brakeReleventSuppCond/3(ESP_MasterCylindBrakePress)
  ESPmasterCylindBrakePressValid/1 -> brakeReleventSuppCond/2(ESP_MasterCylindBrakePressValid)
  Compare To Constant1/1 -> AccReleventSuppCondDelayAbortion/1
  Constant2/1 -> AccReleventSuppCondDelayAbortion/2
  Constant3/1 -> AccReleventSuppCondDelayAbortion/3
  AccrCyclPosnDiagc/1 -> Compare To Constant1/1
  Logical Operator4/1 -> StrReleventSuppCondDelayAbortion/1
  Constant1/1 -> StrReleventSuppCondDelayAbortion/3
  Constant/1 -> StrReleventSuppCondDelayAbortion/2
  Logical Operator5/1 -> cycAEBpartVehRelSuppCondFulfilled/1  "cycAEBpartVehRelSuppCondFulfilled"
  subjVel/1 -> Compare To Constant9/1, Compare To Constant8/1
  Logical Operator/1 -> Logical Operator5/1  "subjVelReleventSuppCondFulfilled"
  Compare To Constant7/1 -> Logical Operator5/5  "vehStateCalcCycAEBPartSuppCondFulfilled"
  Compare To Constant9/1 -> Logical Operator/2
  brakeReleventSuppCond/1(brakeReleventSuppCond) -> Logical Operator5/2  "BrkReleventSuppCondFulfilled"
  StrReleventSuppCondDelayAbortion/1 -> Logical Operator5/3  "strReleventSuppCondFulfilled"
  SteerWheelAngle/1 -> Abs/1
  Abs/1 -> Compare To Constant/1
  Compare To Constant/1 -> Logical Operator4/1
  SteerWheelSpd/1 -> Abs1/1
  Compare To Constant2/1 -> Logical Operator4/2
  Compare To Constant8/1 -> Logical Operator/1
  vehStateCalcCycAEBpartSuppFlg/1 -> Compare To Constant7/1
  Abs1/1 -> Compare To Constant2/1
  AccReleventSuppCondDelayAbortion/1 -> Logical Operator5/4  "accReleventSuppCondFulfilled"

== system TosAeb/FcwAebJa_Step_sys/ALG_AEBSystem/ALG_AEBController/AEB_FuncCondCalcApp/CycFunctionCondCalc/cycPrefillSuppAborCond/cycPrefillAborCondCalc/cycPrefillAborCondFinalJudge ==
  cycPrefillObjRelAborCondFulfilled : Inport
  cycPrefillVehRelAborCondFulfilled : Inport  Port=2
  cycPrefillSituationRelAborCondFulfilled : Inport  Port=3
  Compare To Constant1 : simulink/Logic and Bit Operations/Compare To Constant  relop===  const=TRUE  OutDataTypeStr=boolean
  Compare To Constant2 : simulink/Logic and Bit Operations/Compare To Constant  relop===  const=TRUE  OutDataTypeStr=boolean
  Compare To Constant3 : simulink/Logic and Bit Operations/Compare To Constant  relop===  const=TRUE  OutDataTypeStr=boolean
  Logical Operator : Logic  Operator=OR  Inputs=3  AllPortsSameDT=off  OutDataTypeStr=boolean
  cycPrefillAborCondFulfilled : Outport
  wires:
  Compare To Constant2/1 -> Logical Operator/3
  Compare To Constant1/1 -> Logical Operator/2
  cycPrefillObjRelAborCondFulfilled/1 -> Compare To Constant3/1
  Logical Operator/1 -> cycPrefillAborCondFulfilled/1  "cycPrefillAborCondFulfilled"
  cycPrefillSituationRelAborCondFulfilled/1 -> Compare To Constant2/1
  cycPrefillVehRelAborCondFulfilled/1 -> Compare To Constant1/1
  Compare To Constant3/1 -> Logical Operator/1

== system TosAeb/FcwAebJa_Step_sys/ALG_AEBSystem/ALG_AEBController/AEB_FuncCondCalcApp/CycFunctionCondCalc/cycPrefillSuppAborCond/cycPrefillAborCondCalc/cycPrefillObjRelAborCondCalc ==
  ObjectID : Inport
  Compare To Constant4 : simulink/Logic and Bit Operations/Compare To Constant  relop===  const=CNobjInvalidID  OutDataTypeStr=boolean
  cycPrefillObjRelAborCondFulfilled : Outport
  wires:
  ObjectID/1 -> Compare To Constant4/1
  Compare To Constant4/1 -> cycPrefillObjRelAborCondFulfilled/1

== system TosAeb/FcwAebJa_Step_sys/ALG_AEBSystem/ALG_AEBController/AEB_FuncCondCalcApp/CycFunctionCondCalc/cycPrefillSuppAborCond/cycPrefillAborCondCalc/cycPrefillSituationRelAborCondCalc ==
  note: 更改数值
  CycCollisionSeverity : Inport
  cycPrefillWorkTime : Inport  Port=2
  Compare To Constant : simulink/Logic and Bit Operations/Compare To Constant  relop===  const=CollisionSeverity.None  OutDataTypeStr=boolean
  Compare To Constant1 : simulink/Logic and Bit Operations/Compare To Constant  relop=>=  const=P_AEB_Func_cycPrefillActivationTimeAborThres  OutDataTypeStr=boolean
  Logical Operator : Logic  AllPortsSameDT=off  OutDataTypeStr=boolean
  cycPrefillSituationRelAborCondFulfilled : Outport
  wires:
  CycCollisionSeverity/1 -> Compare To Constant/1
  Compare To Constant/1 -> Logical Operator/1
  Logical Operator/1 -> cycPrefillSituationRelAborCondFulfilled/1
  cycPrefillWorkTime/1 -> Compare To Constant1/1
  Compare To Constant1/1 -> Logical Operator/2

== system TosAeb/FcwAebJa_Step_sys/ALG_AEBSystem/ALG_AEBController/AEB_FuncCondCalcApp/CycFunctionCondCalc/cycPrefillSuppAborCond/cycPrefillAborCondCalc/cycPrefillVehRelAborCondCalc ==
  note: 取消速度限制
  SteerWheelAngle : Inport
  SteerWheelSpd : Inport  Port=2
  vehStateCalcCycPrefillAborFlg : Inport  Port=3
  cycPrefillWorkTime : Inport  Port=4
  Abs : Abs
  Abs1 : Abs
  Compare To Constant : simulink/Logic and Bit Operations/Compare To Constant  relop=>  const=P_AEB_Func_cycPrefillStrWheelAngleAborThres  OutDataTypeStr=boolean
  Compare To Constant1 : simulink/Logic and Bit Operations/Compare To Constant  relop=>  const=P_AEB_Func_cycPrefillStrWheelAngleSpdAborThres  OutDataTypeStr=boolean
  Compare To Constant2 : simulink/Logic and Bit Operations/Compare To Constant  relop=>  const=P_AEB_Func_cycPrefillActivationTimeAborThres  OutDataTypeStr=boolean
  Compare To Constant3 : simulink/Logic and Bit Operations/Compare To Constant  relop===  const=TRUE  OutDataTypeStr=boolean
  Logical Operator : Logic  Operator=OR  AllPortsSameDT=off  OutDataTypeStr=boolean
  Logical Operator2 : Logic  Operator=OR  Inputs=3  AllPortsSameDT=off  OutDataTypeStr=boolean
  cycPrefillVehRelAborCondFulfilled : Outport
  wires:
  Compare To Constant3/1 -> Logical Operator2/2  "vehStateCalcPrefillAborCondFulfilled"
  Abs/1 -> Compare To Constant/1
  SteerWheelSpd/1 -> Abs1/1
  Abs1/1 -> Compare To Constant1/1
  Compare To Constant1/1 -> Logical Operator/2
  SteerWheelAngle/1 -> Abs/1
  cycPrefillWorkTime/1 -> Compare To Constant2/1
  vehStateCalcCycPrefillAborFlg/1 -> Compare To Constant3/1
  Compare To Constant/1 -> Logical Operator/1
  Logical Operator/1 -> Logical Operator2/1  "strReleventAborCondFulfilled"
  Logical Operator2/1 -> cycPrefillVehRelAborCondFulfilled/1
  Compare To Constant2/1 -> Logical Operator2/3  "cycPrefillWorkTimeRelCondFulfilled"

== system TosAeb/FcwAebJa_Step_sys/ALG_AEBSystem/ALG_AEBController/AEB_FuncCondCalcApp/CycFunctionCondCalc/cycPrefillSuppAborCond/cycPrefillSuppCondCalc/cycPrefillObjRelSuppCondCalc ==
  ObjectID : Inport
  vxAbsObj : Inport  Port=2
  ObjFusQuality : Inport  Port=3
  dy : Inport  Port=4
  vy : Inport  Port=5
  Abs : Abs
  Compare To Constant : simulink/Logic and Bit Operations/Compare To Constant  relop=>  const=CNNull  OutDataTypeStr=boolean
  Compare To Constant1 : simulink/Logic and Bit Operations/Compare To Constant  relop=>  const=P_AEB_Func_cycPrefillVxAbsObjSuppThres  OutDataTypeStr=boolean
  Compare To Constant2 : simulink/Logic and Bit Operations/Compare To Constant  relop===  const=FALSE  OutDataTypeStr=boolean
  Compare To Constant3 : simulink/Logic and Bit Operations/Compare To Constant  relop=>  const=CNNull  OutDataTypeStr=boolean
  Compare To Constant4 : simulink/Logic and Bit Operations/Compare To Constant  relop===  const=CNobjInvalidID  OutDataTypeStr=boolean
  Compare To Constant5 : simulink/Logic and Bit Operations/Compare To Constant  relop=<  const=CNNull  OutDataTypeStr=boolean
  Compare To Constant6 : simulink/Logic and Bit Operations/Compare To Constant  relop=<  const=CNNull  OutDataTypeStr=boolean
  Logical Operator : Logic  Operator=OR  Inputs=3  AllPortsSameDT=off  OutDataTypeStr=boolean
  Logical Operator1 : Logic  AllPortsSameDT=off  OutDataTypeStr=boolean
  Logical Operator2 : Logic  AllPortsSameDT=off  OutDataTypeStr=boolean
  Logical Operator3 : Logic  Operator=OR  AllPortsSameDT=off  OutDataTypeStr=boolean
  Terminator : Terminator
  cycPrefillObjRelSuppCondFulfilled : Outport
  wires:
  Compare To Constant2/1 -> Logical Operator/3  "ObjFusQuality"
  ObjFusQuality/1 -> Compare To Constant2/1
  vy/1 -> Compare To Constant3/1, Compare To Constant6/1
  Compare To Constant3/1 -> Logical Operator1/2
  dy/1 -> Compare To Constant/1, Compare To Constant5/1
  Logical Operator2/1 -> Logical Operator3/2
  Compare To Constant/1 -> Logical Operator1/1
  Compare To Constant5/1 -> Logical Operator2/1
  Logical Operator1/1 -> Logical Operator3/1
  Compare To Constant6/1 -> Logical Operator2/2
  Compare To Constant4/1 -> Logical Operator/1
  Logical Operator/1 -> cycPrefillObjRelSuppCondFulfilled/1
  Compare To Constant1/1 -> Logical Operator/2
  Abs/1 -> Compare To Constant1/1
  vxAbsObj/1 -> Abs/1
  ObjectID/1 -> Compare To Constant4/1
  Logical Operator3/1 -> Terminator/1

== system TosAeb/FcwAebJa_Step_sys/ALG_AEBSystem/ALG_AEBController/AEB_FuncCondCalcApp/CycFunctionCondCalc/cycPrefillSuppAborCond/cycPrefillSuppCondCalc/cycPrefillSituationRelSuppCondCalc ==
  latCollisionSeverity : Inport
  subjCurvTraj : Inport  Port=2
  subjLatAccel : Inport  Port=3
  colliisonRiskProb : Inport  Port=4
  ObjectTypeCameraConfirmed : Inport  Port=5
  vy : Inport  Port=6
  dxFrontBumper : Inport  Port=7
  disBraking4PartBrake : Inport  Port=8
  distSecure : Inport  Port=9
  subjVel : Inport  Port=10
  DistBrkCond4PrefillCalc : Subsystem
  cycPrefillSituationRelSuppCondCalc : Subsystem
  objLatVyCond4PrefillCalc : Subsystem
  cycPrefillSituationRelSuppCondFulfilled : Outport
  wires:
  subjVel/1 -> DistBrkCond4PrefillCalc/4(subjVel)
  distSecure/1 -> DistBrkCond4PrefillCalc/3(distSecure)
  disBraking4PartBrake/1 -> DistBrkCond4PrefillCalc/2(disBraking4PartBrake)
  dxFrontBumper/1 -> DistBrkCond4PrefillCalc/1(dxFrontBumper)
  vy/1 -> objLatVyCond4PrefillCalc/2(vy)
  DistBrkCond4PrefillCalc/1(distBrkCond4Prefill) -> cycPrefillSituationRelSuppCondCalc/7(objLongDistCond4Prefill)
  objLatVyCond4PrefillCalc/1(objLatVyCond) -> cycPrefillSituationRelSuppCondCalc/6(objLatVyCond)
  cycPrefillSituationRelSuppCondCalc/1(cycPrefillSituationRelSuppCondFulfilled) -> cycPrefillSituationRelSuppCondFulfilled/1
  ObjectTypeCameraConfirmed/1 -> cycPrefillSituationRelSuppCondCalc/5(ObjectTypeCameraConfirmed), objLatVyCond4PrefillCalc/1(ObjectTypeCameraConfirmed)
  colliisonRiskProb/1 -> cycPrefillSituationRelSuppCondCalc/4(colliisonRiskProb)
  subjLatAccel/1 -> cycPrefillSituationRelSuppCondCalc/3(subjLatAccel)
  subjCurvTraj/1 -> cycPrefillSituationRelSuppCondCalc/2(subjCurvTraj)
  latCollisionSeverity/1 -> cycPrefillSituationRelSuppCondCalc/1(latCollisionSeverity)
